simulink model "HvacCtrlrAirFlowDistbnFrnt"  (R2022b) (continued)
== system HvacCtrlrAirFlowDistbnFrnt == (continued)
  ClimaCfgForClimaCtrlMan1_ClimaCfgForClimaCtrlMan1/1 -> HvacCtrlrAirFlowDistbnFrnt_Runnable_sys/26(ClimaCfgForClimaCtrlMan1_ClimaCfgForClimaCtrlMan1_read)
  ClimaCfgForNrOfTSetg1_ClimaCfgForNrOfTSetg1/1 -> HvacCtrlrAirFlowDistbnFrnt_Runnable_sys/27(ClimaCfgForNrOfTSetg1_ClimaCfgForNrOfTSetg1_read)
  ClimaCtrlActvNgcc_ClimaCtrlActv/1 -> HvacCtrlrAirFlowDistbnFrnt_Runnable_sys/28(ClimaCtrlActvNgcc_ClimaCtrlActv_read)
  HmiCmptmtAirDistbnFrntForClima_HmiCmptmtAirDistbnFrntForClima/1 -> HvacCtrlrAirFlowDistbnFrnt_Runnable_sys/29(HmiCmptmtAirDistbnFrntForClima_HmiCmptmtAirDistbnFrntForClima_read)
  HmiCmptmtAirDistbnFrntForClima_HmiCmptmtAirDistbnFrntRiForClima/1 -> HvacCtrlrAirFlowDistbnFrnt_Runnable_sys/30(HmiCmptmtAirDistbnFrntForClima_HmiCmptmtAirDistbnFrntRiForClima_read)
  HmiCmptmtAirDistbnFrntForClima_HmiCmptmtAirDistbnReForClima/1 -> HvacCtrlrAirFlowDistbnFrnt_Runnable_sys/31(HmiCmptmtAirDistbnFrntForClima_HmiCmptmtAirDistbnReForClima_read)
  HmiCmptmtAirDistbnFrntForClima_HmiCmptmtAirDistbnReRiForClima/1 -> HvacCtrlrAirFlowDistbnFrnt_Runnable_sys/32(HmiCmptmtAirDistbnFrntForClima_HmiCmptmtAirDistbnReRiForClima_read)
  HvacAirMFlowEstimdForCtrlr_HvacAirMFlowEstimdForCtrlr/1 -> HvacCtrlrAirFlowDistbnFrnt_Runnable_sys/33(HvacAirMFlowEstimdForCtrlr_HvacAirMFlowEstimdForCtrlr_read)
  HvacCtrlrFanBoostReqLe_HvacCtrlrFanBoostReqLe/1 -> HvacCtrlrAirFlowDistbnFrnt_Runnable_sys/34(HvacCtrlrFanBoostReqLe_HvacCtrlrFanBoostReqLe_read)
  HvacCtrlrFanBoostReqRi_HvacCtrlrFanBoostReqRi/1 -> HvacCtrlrAirFlowDistbnFrnt_Runnable_sys/35(HvacCtrlrFanBoostReqRi_HvacCtrlrFanBoostReqRi_read)
  HvacCtrlrReqFrntForRowFirstForActrTLe_HvacCtrlrReqFrntForRowFirstForActrTLe/1 -> HvacCtrlrAirFlowDistbnFrnt_Runnable_sys/36(HvacCtrlrReqFrntForRowFirstForActrTLe_HvacCtrlrReqFrntForRowFirstForActrTLe_read)
  HvacCtrlrReqFrntForRowFirstForActrTRi_HvacCtrlrReqFrntForRowFirstForActrTRi/1 -> HvacCtrlrAirFlowDistbnFrnt_Runnable_sys/37(HvacCtrlrReqFrntForRowFirstForActrTRi_HvacCtrlrReqFrntForRowFirstForActrTRi_read)
  HvacCtrlrReqFrntForRowSecForActrTLe_HvacCtrlrReqFrntForRowSecForActrTLe/1 -> HvacCtrlrAirFlowDistbnFrnt_Runnable_sys/38(HvacCtrlrReqFrntForRowSecForActrTLe_HvacCtrlrReqFrntForRowSecForActrTLe_read)
  HvacCtrlrReqFrntForRowSecForActrTRi_HvacCtrlrReqFrntForRowSecForActrTRi/1 -> HvacCtrlrAirFlowDistbnFrnt_Runnable_sys/39(HvacCtrlrReqFrntForRowSecForActrTRi_HvacCtrlrReqFrntForRowSecForActrTRi_read)
  HvacFlowFrntActForDefrst_HvacFlowFrntActForDefrst/1 -> HvacCtrlrAirFlowDistbnFrnt_Runnable_sys/40(HvacFlowFrntActForDefrst_HvacFlowFrntActForDefrst_read)
  HvacFlowFrntActForRowFirstAtFlr_HvacFlowFrntActForRowFirstAtFlr/1 -> HvacCtrlrAirFlowDistbnFrnt_Runnable_sys/41(HvacFlowFrntActForRowFirstAtFlr_HvacFlowFrntActForRowFirstAtFlr_read)
  HvacFlowFrntActForRowFirstAtFlr_HvacFlowFrntActForRowFirstAtFlrLe/1 -> HvacCtrlrAirFlowDistbnFrnt_Runnable_sys/42(HvacFlowFrntActForRowFirstAtFlr_HvacFlowFrntActForRowFirstAtFlrLe_read)
  HvacFlowFrntActForRowFirstAtFlr_HvacFlowFrntActForRowFirstAtFlrRi/1 -> HvacCtrlrAirFlowDistbnFrnt_Runnable_sys/43(HvacFlowFrntActForRowFirstAtFlr_HvacFlowFrntActForRowFirstAtFlrRi_read)
  HvacFlowFrntActForRowFirstAtVent_HvacFlowFrntActForRowFirstAtVent/1 -> HvacCtrlrAirFlowDistbnFrnt_Runnable_sys/44(HvacFlowFrntActForRowFirstAtVent_HvacFlowFrntActForRowFirstAtVent_read)
  HvacFlowFrntActForRowFirstAtVent_HvacFlowFrntActForRowFirstAtVentLe/1 -> HvacCtrlrAirFlowDistbnFrnt_Runnable_sys/45(HvacFlowFrntActForRowFirstAtVent_HvacFlowFrntActForRowFirstAtVentLe_read)
  HvacFlowFrntActForRowFirstAtVent_HvacFlowFrntActForRowFirstAtVentRi/1 -> HvacCtrlrAirFlowDistbnFrnt_Runnable_sys/46(HvacFlowFrntActForRowFirstAtVent_HvacFlowFrntActForRowFirstAtVentRi_read)
  HvacFlowFrntActForRowFirstAtVent_HvacFlowFrntActForRowFirstAtVentSideLe/1 -> HvacCtrlrAirFlowDistbnFrnt_Runnable_sys/47(HvacFlowFrntActForRowFirstAtVent_HvacFlowFrntActForRowFirstAtVentSideLe_read)
  HvacFlowFrntActForRowFirstAtVent_HvacFlowFrntActForRowFirstAtVentSideRi/1 -> HvacCtrlrAirFlowDistbnFrnt_Runnable_sys/48(HvacFlowFrntActForRowFirstAtVent_HvacFlowFrntActForRowFirstAtVentSideRi_read)
  HvacFlowFrntActForRowSecAtFlr_HvacFlowFrntActForRowSecAtFlr/1 -> HvacCtrlrAirFlowDistbnFrnt_Runnable_sys/49(HvacFlowFrntActForRowSecAtFlr_HvacFlowFrntActForRowSecAtFlr_read)
  HvacFlowFrntActForRowSecAtFlr_HvacFlowFrntActForRowSecAtFlrLe/1 -> HvacCtrlrAirFlowDistbnFrnt_Runnable_sys/50(HvacFlowFrntActForRowSecAtFlr_HvacFlowFrntActForRowSecAtFlrLe_read)
  HvacFlowFrntActForRowSecAtFlr_HvacFlowFrntActForRowSecAtFlrRi/1 -> HvacCtrlrAirFlowDistbnFrnt_Runnable_sys/51(HvacFlowFrntActForRowSecAtFlr_HvacFlowFrntActForRowSecAtFlrRi_read)
  HvacFlowFrntActForRowSecAtVent_HvacFlowFrntActForRowSecAtVent/1 -> HvacCtrlrAirFlowDistbnFrnt_Runnable_sys/52(HvacFlowFrntActForRowSecAtVent_HvacFlowFrntActForRowSecAtVent_read)
  HvacFlowFrntActForRowSecAtVent_HvacFlowFrntActForRowSecAtVentLe/1 -> HvacCtrlrAirFlowDistbnFrnt_Runnable_sys/53(HvacFlowFrntActForRowSecAtVent_HvacFlowFrntActForRowSecAtVentLe_read)
  HvacFlowFrntActForRowSecAtVent_HvacFlowFrntActForRowSecAtVentRi/1 -> HvacCtrlrAirFlowDistbnFrnt_Runnable_sys/54(HvacFlowFrntActForRowSecAtVent_HvacFlowFrntActForRowSecAtVentRi_read)
  HvacFlowFrntReqForDefrst_HvacFlowFrntReqForDefrst/1 -> HvacCtrlrAirFlowDistbnFrnt_Runnable_sys/55(HvacFlowFrntReqForDefrst_HvacFlowFrntReqForDefrst_read)
  HvacFlowFrntReqForRowFirstAtFlr_HvacFlowFrntReqForRowFirstAtFlr/1 -> HvacCtrlrAirFlowDistbnFrnt_Runnable_sys/56(HvacFlowFrntReqForRowFirstAtFlr_HvacFlowFrntReqForRowFirstAtFlr_read)
  HvacFlowFrntReqForRowFirstAtFlr_HvacFlowFrntReqForRowFirstAtFlrLe/1 -> HvacCtrlrAirFlowDistbnFrnt_Runnable_sys/57(HvacFlowFrntReqForRowFirstAtFlr_HvacFlowFrntReqForRowFirstAtFlrLe_read)
  HvacFlowFrntReqForRowFirstAtFlr_HvacFlowFrntReqForRowFirstAtFlrRi/1 -> HvacCtrlrAirFlowDistbnFrnt_Runnable_sys/58(HvacFlowFrntReqForRowFirstAtFlr_HvacFlowFrntReqForRowFirstAtFlrRi_read)
  HvacFlowFrntReqForRowFirstAtVent_HvacFlowFrntReqForRowFirstAtVent/1 -> HvacCtrlrAirFlowDistbnFrnt_Runnable_sys/59(HvacFlowFrntReqForRowFirstAtVent_HvacFlowFrntReqForRowFirstAtVent_read)
  HvacFlowFrntReqForRowFirstAtVent_HvacFlowFrntReqForRowFirstAtVentLe/1 -> HvacCtrlrAirFlowDistbnFrnt_Runnable_sys/60(HvacFlowFrntReqForRowFirstAtVent_HvacFlowFrntReqForRowFirstAtVentLe_read)
  HvacFlowFrntReqForRowFirstAtVent_HvacFlowFrntReqForRowFirstAtVentLeMid/1 -> HvacCtrlrAirFlowDistbnFrnt_Runnable_sys/61(HvacFlowFrntReqForRowFirstAtVent_HvacFlowFrntReqForRowFirstAtVentLeMid_read)
  HvacFlowFrntReqForRowFirstAtVent_HvacFlowFrntReqForRowFirstAtVentLeSide/1 -> HvacCtrlrAirFlowDistbnFrnt_Runnable_sys/62(HvacFlowFrntReqForRowFirstAtVent_HvacFlowFrntReqForRowFirstAtVentLeSide_read)
  HvacFlowFrntReqForRowFirstAtVent_HvacFlowFrntReqForRowFirstAtVentRi/1 -> HvacCtrlrAirFlowDistbnFrnt_Runnable_sys/63(HvacFlowFrntReqForRowFirstAtVent_HvacFlowFrntReqForRowFirstAtVentRi_read)
  HvacFlowFrntReqForRowFirstAtVent_HvacFlowFrntReqForRowFirstAtVentRiMid/1 -> HvacCtrlrAirFlowDistbnFrnt_Runnable_sys/64(HvacFlowFrntReqForRowFirstAtVent_HvacFlowFrntReqForRowFirstAtVentRiMid_read)
  HvacFlowFrntReqForRowFirstAtVent_HvacFlowFrntReqForRowFirstAtVentRiSide/1 -> HvacCtrlrAirFlowDistbnFrnt_Runnable_sys/65(HvacFlowFrntReqForRowFirstAtVent_HvacFlowFrntReqForRowFirstAtVentRiSide_read)
  HvacFlowFrntReqForRowSecAtFlr_HvacFlowFrntReqForRowSecAtFlr/1 -> HvacCtrlrAirFlowDistbnFrnt_Runnable_sys/66(HvacFlowFrntReqForRowSecAtFlr_HvacFlowFrntReqForRowSecAtFlr_read)
  HvacFlowFrntReqForRowSecAtFlr_HvacFlowFrntReqForRowSecAtFlrLe/1 -> HvacCtrlrAirFlowDistbnFrnt_Runnable_sys/67(HvacFlowFrntReqForRowSecAtFlr_HvacFlowFrntReqForRowSecAtFlrLe_read)
  HvacFlowFrntReqForRowSecAtFlr_HvacFlowFrntReqForRowSecAtFlrRi/1 -> HvacCtrlrAirFlowDistbnFrnt_Runnable_sys/68(HvacFlowFrntReqForRowSecAtFlr_HvacFlowFrntReqForRowSecAtFlrRi_read)
  HvacFlowFrntReqForRowSecAtVent_HvacFlowFrntReqForRowSecAtVent/1 -> HvacCtrlrAirFlowDistbnFrnt_Runnable_sys/69(HvacFlowFrntReqForRowSecAtVent_HvacFlowFrntReqForRowSecAtVent_read)
  HvacFlowFrntReqForRowSecAtVent_HvacFlowFrntReqForRowSecAtVentLe/1 -> HvacCtrlrAirFlowDistbnFrnt_Runnable_sys/70(HvacFlowFrntReqForRowSecAtVent_HvacFlowFrntReqForRowSecAtVentLe_read)
  HvacFlowFrntReqForRowSecAtVent_HvacFlowFrntReqForRowSecAtVentRi/1 -> HvacCtrlrAirFlowDistbnFrnt_Runnable_sys/71(HvacFlowFrntReqForRowSecAtVent_HvacFlowFrntReqForRowSecAtVentRi_read)
  HvacFlrLeakReqForRowFirst_HvacFlrLeakReqForRowFirst/1 -> HvacCtrlrAirFlowDistbnFrnt_Runnable_sys/72(HvacFlrLeakReqForRowFirst_HvacFlrLeakReqForRowFirst_read)
  HvacFlrLeakReqForRowSec_HvacFlrLeakReqForRowSec/1 -> HvacCtrlrAirFlowDistbnFrnt_Runnable_sys/73(HvacFlrLeakReqForRowSec_HvacFlrLeakReqForRowSec_read)
  LdAdVal_CurrAdVal/1 -> HvacCtrlrAirFlowDistbnFrnt_Runnable_sys/74(LdAdVal_CurrAdVal_read)
  LdAdVal_ValidAdVal/1 -> HvacCtrlrAirFlowDistbnFrnt_Runnable_sys/75(LdAdVal_ValidAdVal_read)
  ManModDurgFanOffActv_ManModDurgFanOffActv/1 -> HvacCtrlrAirFlowDistbnFrnt_Runnable_sys/76(ManModDurgFanOffActv_ManModDurgFanOffActv_read)
  ManModeForRowFirstLeForModelDial_ManModeForRowFirstLeForModelDial/1 -> HvacCtrlrAirFlowDistbnFrnt_Runnable_sys/77(ManModeForRowFirstLeForModelDial_ManModeForRowFirstLeForModelDial_read)
  ManModeForRowFirstRiForModelDial_ManModeForRowFirstRiForModelDial/1 -> HvacCtrlrAirFlowDistbnFrnt_Runnable_sys/78(ManModeForRowFirstRiForModelDial_ManModeForRowFirstRiForModelDial_read)
  ManModeForRowSecLeForModelDial_ManModeForRowSecLeForModelDial/1 -> HvacCtrlrAirFlowDistbnFrnt_Runnable_sys/79(ManModeForRowSecLeForModelDial_ManModeForRowSecLeForModelDial_read)
  ManModeForRowSecRiForModelDial_ManModeForRowSecRiForModelDial/1 -> HvacCtrlrAirFlowDistbnFrnt_Runnable_sys/80(ManModeForRowSecRiForModelDial_ManModeForRowSecRiForModelDial_read)
  MaxReqReqdFrntForDefrst_MaxReqReqdFrntForDefrst/1 -> HvacCtrlrAirFlowDistbnFrnt_Runnable_sys/81(MaxReqReqdFrntForDefrst_MaxReqReqdFrntForDefrst_read)
  MaxReqReqdFrntForRowFirstAtFlr_MaxReqReqdFrntForRowFirstAtFlr/1 -> HvacCtrlrAirFlowDistbnFrnt_Runnable_sys/82(MaxReqReqdFrntForRowFirstAtFlr_MaxReqReqdFrntForRowFirstAtFlr_read)
  MaxReqReqdFrntForRowFirstAtFlr_MaxReqReqdFrntForRowFirstAtFlrLe/1 -> HvacCtrlrAirFlowDistbnFrnt_Runnable_sys/83(MaxReqReqdFrntForRowFirstAtFlr_MaxReqReqdFrntForRowFirstAtFlrLe_read)
  MaxReqReqdFrntForRowFirstAtFlr_MaxReqReqdFrntForRowFirstAtFlrRi/1 -> HvacCtrlrAirFlowDistbnFrnt_Runnable_sys/84(MaxReqReqdFrntForRowFirstAtFlr_MaxReqReqdFrntForRowFirstAtFlrRi_read)
  MaxReqReqdFrntForRowFirstAtVent_MaxReqReqdFrntForRowFirstAtVent/1 -> HvacCtrlrAirFlowDistbnFrnt_Runnable_sys/85(MaxReqReqdFrntForRowFirstAtVent_MaxReqReqdFrntForRowFirstAtVent_read)
  MaxReqReqdFrntForRowFirstAtVent_MaxReqReqdFrntForRowFirstAtVentLe/1 -> HvacCtrlrAirFlowDistbnFrnt_Runnable_sys/86(MaxReqReqdFrntForRowFirstAtVent_MaxReqReqdFrntForRowFirstAtVentLe_read)
  MaxReqReqdFrntForRowFirstAtVent_MaxReqReqdFrntForRowFirstAtVentRi/1 -> HvacCtrlrAirFlowDistbnFrnt_Runnable_sys/87(MaxReqReqdFrntForRowFirstAtVent_MaxReqReqdFrntForRowFirstAtVentRi_read)
  MaxReqReqdFrntForRowFirstAtVent_MaxReqReqdFrntForRowFirstAtVentSideLe/1 -> HvacCtrlrAirFlowDistbnFrnt_Runnable_sys/88(MaxReqReqdFrntForRowFirstAtVent_MaxReqReqdFrntForRowFirstAtVentSideLe_read)
  MaxReqReqdFrntForRowFirstAtVent_MaxReqReqdFrntForRowFirstAtVentSideRi/1 -> HvacCtrlrAirFlowDistbnFrnt_Runnable_sys/89(MaxReqReqdFrntForRowFirstAtVent_MaxReqReqdFrntForRowFirstAtVentSideRi_read)
  MaxReqReqdFrntForRowSecAtFlr_MaxReqReqdFrntForRowSecAtFlr/1 -> HvacCtrlrAirFlowDistbnFrnt_Runnable_sys/90(MaxReqReqdFrntForRowSecAtFlr_MaxReqReqdFrntForRowSecAtFlr_read)
  MaxReqReqdFrntForRowSecAtFlr_MaxReqReqdFrntForRowSecAtFlrLe/1 -> HvacCtrlrAirFlowDistbnFrnt_Runnable_sys/91(MaxReqReqdFrntForRowSecAtFlr_MaxReqReqdFrntForRowSecAtFlrLe_read)
  MaxReqReqdFrntForRowSecAtFlr_MaxReqReqdFrntForRowSecAtFlrRi/1 -> HvacCtrlrAirFlowDistbnFrnt_Runnable_sys/92(MaxReqReqdFrntForRowSecAtFlr_MaxReqReqdFrntForRowSecAtFlrRi_read)
  MaxReqReqdFrntForRowSecAtVent_MaxReqReqdFrntForRowSecAtVent/1 -> HvacCtrlrAirFlowDistbnFrnt_Runnable_sys/93(MaxReqReqdFrntForRowSecAtVent_MaxReqReqdFrntForRowSecAtVent_read)
  MaxReqReqdFrntForRowSecAtVent_MaxReqReqdFrntForRowSecAtVentLe/1 -> HvacCtrlrAirFlowDistbnFrnt_Runnable_sys/94(MaxReqReqdFrntForRowSecAtVent_MaxReqReqdFrntForRowSecAtVentLe_read)
  MaxReqReqdFrntForRowSecAtVent_MaxReqReqdFrntForRowSecAtVentRi/1 -> HvacCtrlrAirFlowDistbnFrnt_Runnable_sys/95(MaxReqReqdFrntForRowSecAtVent_MaxReqReqdFrntForRowSecAtVentRi_read)
  NotAllwdForMaxReqReqdForRowSecAtVent_NotAllwdForMaxReqReqdForRowSecAtVent/1 -> HvacCtrlrAirFlowDistbnFrnt_Runnable_sys/96(NotAllwdForMaxReqReqdForRowSecAtVent_NotAllwdForMaxReqReqdForRowSecAtVent_read)
  VehSpdLgtForClima_VehSpdLgtForClima/1 -> HvacCtrlrAirFlowDistbnFrnt_Runnable_sys/97(VehSpdLgtForClima_VehSpdLgtForClima_read)
  VehSunLoadAtRowFirstLeFild_VehSunLoadAtRowFirstLeFild/1 -> HvacCtrlrAirFlowDistbnFrnt_Runnable_sys/98(VehSunLoadAtRowFirstLeFild_VehSunLoadAtRowFirstLeFild_read)
  VehSunLoadAtRowFirstRiFild_VehSunLoadAtRowFirstRiFild/1 -> HvacCtrlrAirFlowDistbnFrnt_Runnable_sys/99(VehSunLoadAtRowFirstRiFild_VehSunLoadAtRowFirstRiFild_read)
  ActrFrntReqForRowFirstLeModDistbn_ActrFrntReqForRowFirstLeModDistbn/1 -> HvacCtrlrAirFlowDistbnFrnt_Runnable_sys/100(ActrFrntReqForRowFirstLeModDistbn_ActrFrntReqForRowFirstLeModDistbn_read)
  ActrFrntReqForRowFirstLeModDistbn_ActrFrntReqForRowFirstLeModDistbn_IsUpdated/1 -> HvacCtrlrAirFlowDistbnFrnt_Runnable_sys/101(ActrFrntReqForRowFirstLeModDistbn_ActrFrntReqForRowFirstLeModDistbn_IsUpdated_read)
  ActrFrntReqForRowFirstModDistbn_ActrFrntReqForRowFirstModDistbn/1 -> HvacCtrlrAirFlowDistbnFrnt_Runnable_sys/102(ActrFrntReqForRowFirstModDistbn_ActrFrntReqForRowFirstModDistbn_read)
  ActrFrntReqForRowFirstModDistbn_ActrFrntReqForRowFirstModDistbn_IsUpdated/1 -> HvacCtrlrAirFlowDistbnFrnt_Runnable_sys/103(ActrFrntReqForRowFirstModDistbn_ActrFrntReqForRowFirstModDistbn_IsUpdated_read)
  ActrFrntReqForRowSecModDistbnLe_ActrFrntReqForRowSecModDistbnLe/1 -> HvacCtrlrAirFlowDistbnFrnt_Runnable_sys/104(ActrFrntReqForRowSecModDistbnLe_ActrFrntReqForRowSecModDistbnLe_read)
  ActrFrntReqForRowSecModDistbnLe_ActrFrntReqForRowSecModDistbnLe_IsUpdated/1 -> HvacCtrlrAirFlowDistbnFrnt_Runnable_sys/105(ActrFrntReqForRowSecModDistbnLe_ActrFrntReqForRowSecModDistbnLe_IsUpdated_read)
  ActrFrntReqForRowSecModDistbnRi_ActrFrntReqForRowSecModDistbnRi/1 -> HvacCtrlrAirFlowDistbnFrnt_Runnable_sys/106(ActrFrntReqForRowSecModDistbnRi_ActrFrntReqForRowSecModDistbnRi_read)
  ActrFrntReqForRowSecModDistbnRi_ActrFrntReqForRowSecModDistbnRi_IsUpdated/1 -> HvacCtrlrAirFlowDistbnFrnt_Runnable_sys/107(ActrFrntReqForRowSecModDistbnRi_ActrFrntReqForRowSecModDistbnRi_IsUpdated_read)
  ActrPosnFrntActForRowFirstModDistbnLe_ActrPosnFrntActForRowFirstModDistbnLe/1 -> HvacCtrlrAirFlowDistbnFrnt_Runnable_sys/108(ActrPosnFrntActForRowFirstModDistbnLe_ActrPosnFrntActForRowFirstModDistbnLe_read)
  ActrReqForModDistbnRe_ActrReqForModDistbnRe/1 -> HvacCtrlrAirFlowDistbnFrnt_Runnable_sys/109(ActrReqForModDistbnRe_ActrReqForModDistbnRe_read)
  ActrReqForModDistbnRe_ActrReqForModDistbnRe_IsUpdated/1 -> HvacCtrlrAirFlowDistbnFrnt_Runnable_sys/110(ActrReqForModDistbnRe_ActrReqForModDistbnRe_IsUpdated_read)
  ClimaAtRowSecActvNgcc_ClimaAtRowSecActv/1 -> HvacCtrlrAirFlowDistbnFrnt_Runnable_sys/111(ClimaAtRowSecActvNgcc_ClimaAtRowSecActv_read)
  ClimaAtRowSecActvNgcc_ClimaAtRowSecActvLe/1 -> HvacCtrlrAirFlowDistbnFrnt_Runnable_sys/112(ClimaAtRowSecActvNgcc_ClimaAtRowSecActvLe_read)
  ClimaAtRowSecActvNgcc_ClimaAtRowSecActvRi/1 -> HvacCtrlrAirFlowDistbnFrnt_Runnable_sys/113(ClimaAtRowSecActvNgcc_ClimaAtRowSecActvRi_read)
  ClimaCfgForHvacRePrsnt1_ClimaCfgForHvacRePrsnt1/1 -> HvacCtrlrAirFlowDistbnFrnt_Runnable_sys/114(ClimaCfgForHvacRePrsnt1_ClimaCfgForHvacRePrsnt1_read)
  ClimaCfgReqVersionReplace_ClimaCfgReqVersionReplace/1 -> HvacCtrlrAirFlowDistbnFrnt_Runnable_sys/115(ClimaCfgReqVersionReplace_ClimaCfgReqVersionReplace_read)
  ManModeForRowThrdForModelDial_ManModeForRowThrdForModelDial/1 -> HvacCtrlrAirFlowDistbnFrnt_Runnable_sys/116(ManModeForRowThrdForModelDial_ManModeForRowThrdForModelDial_read)
  PrkgClimaOnReq_PrkgClimaOnReq/1 -> HvacCtrlrAirFlowDistbnFrnt_Runnable_sys/117(PrkgClimaOnReq_PrkgClimaOnReq_read)
  HvacCtrlrAirFlowDistbnFrnt_Runnable_sys/1(HvacAirMFlowReqMaxAvl_HvacAirMFlowReqMaxAvl_write) -> HvacAirMFlowReqMaxAvl_HvacAirMFlowReqMaxAvl/1
  HvacCtrlrAirFlowDistbnFrnt_Runnable_sys/2(HvacCtrlrReqFrntForRowFirstForActrDefrst_HvacCtrlrReqFrntForRowFirstForActrDefrst_write) -> HvacCtrlrReqFrntForRowFirstForActrDefrst_HvacCtrlrReqFrntForRowFirstForActrDefrst/1
  HvacCtrlrAirFlowDistbnFrnt_Runnable_sys/3(HvacCtrlrReqFrntForRowFirstForActrFlr_HvacCtrlrReqFrntForRowFirstForActrFlr_write) -> HvacCtrlrReqFrntForRowFirstForActrFlr_HvacCtrlrReqFrntForRowFirstForActrFlr/1
  HvacCtrlrAirFlowDistbnFrnt_Runnable_sys/4(HvacCtrlrReqFrntForRowFirstForActrFlr_HvacCtrlrReqFrntForRowFirstForActrFlrLe_write) -> HvacCtrlrReqFrntForRowFirstForActrFlr_HvacCtrlrReqFrntForRowFirstForActrFlrLe/1
  HvacCtrlrAirFlowDistbnFrnt_Runnable_sys/5(HvacCtrlrReqFrntForRowFirstForActrFlr_HvacCtrlrReqFrntForRowFirstForActrFlrRi_write) -> HvacCtrlrReqFrntForRowFirstForActrFlr_HvacCtrlrReqFrntForRowFirstForActrFlrRi/1
  HvacCtrlrAirFlowDistbnFrnt_Runnable_sys/6(HvacCtrlrReqFrntForRowFirstForActrMod_HvacCtrlrReqFrntForRowFirstForActrMod_write) -> HvacCtrlrReqFrntForRowFirstForActrMod_HvacCtrlrReqFrntForRowFirstForActrMod/1
  HvacCtrlrAirFlowDistbnFrnt_Runnable_sys/7(HvacCtrlrReqFrntForRowFirstForActrVent_HvacCtrlrReqFrntForRowFirstForActrVent_write) -> HvacCtrlrReqFrntForRowFirstForActrVent_HvacCtrlrReqFrntForRowFirstForActrVent/1
  HvacCtrlrAirFlowDistbnFrnt_Runnable_sys/8(HvacCtrlrReqFrntForRowFirstForActrVent_HvacCtrlrReqFrntForRowFirstForActrVentLe_write) -> HvacCtrlrReqFrntForRowFirstForActrVent_HvacCtrlrReqFrntForRowFirstForActrVentLe/1
  HvacCtrlrAirFlowDistbnFrnt_Runnable_sys/9(HvacCtrlrReqFrntForRowFirstForActrVent_HvacCtrlrReqFrntForRowFirstForActrVentRi_write) -> HvacCtrlrReqFrntForRowFirstForActrVent_HvacCtrlrReqFrntForRowFirstForActrVentRi/1
  HvacCtrlrAirFlowDistbnFrnt_Runnable_sys/10(HvacCtrlrReqFrntForRowFirstForActrVent_HvacCtrlrReqFrntForRowFirstForActrVentSideLe_write) -> HvacCtrlrReqFrntForRowFirstForActrVent_HvacCtrlrReqFrntForRowFirstForActrVentSideLe/1
  HvacCtrlrAirFlowDistbnFrnt_Runnable_sys/11(HvacCtrlrReqFrntForRowFirstForActrVent_HvacCtrlrReqFrntForRowFirstForActrVentSideRi_write) -> HvacCtrlrReqFrntForRowFirstForActrVent_HvacCtrlrReqFrntForRowFirstForActrVentSideRi/1
  HvacCtrlrAirFlowDistbnFrnt_Runnable_sys/12(HvacCtrlrReqFrntForRowFirstLeForActrMod_HvacCtrlrReqFrntForRowFirstLeForActrMod_write) -> HvacCtrlrReqFrntForRowFirstLeForActrMod_HvacCtrlrReqFrntForRowFirstLeForActrMod/1
  HvacCtrlrAirFlowDistbnFrnt_Runnable_sys/13(HvacCtrlrReqFrntForRowSecForActrFlr_HvacCtrlrReqFrntForRowSecForActrFlr_write) -> HvacCtrlrReqFrntForRowSecForActrFlr_HvacCtrlrReqFrntForRowSecForActrFlr/1
  HvacCtrlrAirFlowDistbnFrnt_Runnable_sys/14(HvacCtrlrReqFrntForRowSecForActrFlr_HvacCtrlrReqFrntForRowSecForActrFlrLe_write) -> HvacCtrlrReqFrntForRowSecForActrFlr_HvacCtrlrReqFrntForRowSecForActrFlrLe/1
  HvacCtrlrAirFlowDistbnFrnt_Runnable_sys/15(HvacCtrlrReqFrntForRowSecForActrFlr_HvacCtrlrReqFrntForRowSecForActrFlrRi_write) -> HvacCtrlrReqFrntForRowSecForActrFlr_HvacCtrlrReqFrntForRowSecForActrFlrRi/1
  HvacCtrlrAirFlowDistbnFrnt_Runnable_sys/16(HvacCtrlrReqFrntForRowSecForActrModLe_HvacCtrlrReqFrntForRowSecForActrModLe_write) -> HvacCtrlrReqFrntForRowSecForActrModLe_HvacCtrlrReqFrntForRowSecForActrModLe/1
  HvacCtrlrAirFlowDistbnFrnt_Runnable_sys/17(HvacCtrlrReqFrntForRowSecForActrModRi_HvacCtrlrReqFrntForRowSecForActrModRi_write) -> HvacCtrlrReqFrntForRowSecForActrModRi_HvacCtrlrReqFrntForRowSecForActrModRi/1
  HvacCtrlrAirFlowDistbnFrnt_Runnable_sys/18(HvacCtrlrReqFrntForRowSecForActrVent_HvacCtrlrReqFrntForRowSecForActrVent_write) -> HvacCtrlrReqFrntForRowSecForActrVent_HvacCtrlrReqFrntForRowSecForActrVent/1
  HvacCtrlrAirFlowDistbnFrnt_Runnable_sys/19(OvrdForMaxReqForActrForDefrst_OvrdForMaxReqForActrForDefrst_write) -> OvrdForMaxReqForActrForDefrst_OvrdForMaxReqForActrForDefrst/1
  HvacCtrlrAirFlowDistbnFrnt_Runnable_sys/20(OvrdForMaxReqForActrForRowFirstAtFlr_OvrdForMaxReqForActrForRowFirstAtFlr_write) -> OvrdForMaxReqForActrForRowFirstAtFlr_OvrdForMaxReqForActrForRowFirstAtFlr/1
  OvrdForMaxReqForActrForRowFirstAtFlr_OvrdForMaxReqForActrForRowFirstAtFlrLe_merge/1 -> OvrdForMaxReqForActrForRowFirstAtFlr_OvrdForMaxReqForActrForRowFirstAtFlrLe/1
  OvrdForMaxReqForActrForRowFirstAtFlr_OvrdForMaxReqForActrForRowFirstAtFlrRi_merge/1 -> OvrdForMaxReqForActrForRowFirstAtFlr_OvrdForMaxReqForActrForRowFirstAtFlrRi/1
  HvacCtrlrAirFlowDistbnFrnt_Runnable_sys/23(OvrdForMaxReqForActrForRowFirstAtVent_OvrdForMaxReqForActrForRowFirstAtVent_write) -> OvrdForMaxReqForActrForRowFirstAtVent_OvrdForMaxReqForActrForRowFirstAtVent/1
  OvrdForMaxReqForActrForRowFirstAtVent_OvrdForMaxReqForActrForRowFirstAtVentLe_merge/1 -> OvrdForMaxReqForActrForRowFirstAtVent_OvrdForMaxReqForActrForRowFirstAtVentLe/1
  OvrdForMaxReqForActrForRowFirstAtVent_OvrdForMaxReqForActrForRowFirstAtVentRi_merge/1 -> OvrdForMaxReqForActrForRowFirstAtVent_OvrdForMaxReqForActrForRowFirstAtVentRi/1
  OvrdForMaxReqForActrForRowFirstAtVent_OvrdForMaxReqForActrForRowFirstAtVentSideLe_merge/1 -> OvrdForMaxReqForActrForRowFirstAtVent_OvrdForMaxReqForActrForRowFirstAtVentSideLe/1
  OvrdForMaxReqForActrForRowFirstAtVent_OvrdForMaxReqForActrForRowFirstAtVentSideRi_merge/1 -> OvrdForMaxReqForActrForRowFirstAtVent_OvrdForMaxReqForActrForRowFirstAtVentSideRi/1
  HvacCtrlrAirFlowDistbnFrnt_Runnable_sys/28(OvrdForMaxReqForActrForRowSecAtFlr_OvrdForMaxReqForActrForRowSecAtFlr_write) -> OvrdForMaxReqForActrForRowSecAtFlr_OvrdForMaxReqForActrForRowSecAtFlr/1
  OvrdForMaxReqForActrForRowSecAtFlr_OvrdForMaxReqForActrForRowSecAtFlrLe_merge/1 -> OvrdForMaxReqForActrForRowSecAtFlr_OvrdForMaxReqForActrForRowSecAtFlrLe/1
  OvrdForMaxReqForActrForRowSecAtFlr_OvrdForMaxReqForActrForRowSecAtFlrRi_merge/1 -> OvrdForMaxReqForActrForRowSecAtFlr_OvrdForMaxReqForActrForRowSecAtFlrRi/1
  HvacCtrlrAirFlowDistbnFrnt_Runnable_sys/31(OvrdForMaxReqForActrForRowSecAtVent_OvrdForMaxReqForActrForRowSecAtVent_write) -> OvrdForMaxReqForActrForRowSecAtVent_OvrdForMaxReqForActrForRowSecAtVent/1
  OvrdForMaxReqForActrForRowSecAtVent_OvrdForMaxReqForActrForRowSecAtVentLe_merge/1 -> OvrdForMaxReqForActrForRowSecAtVent_OvrdForMaxReqForActrForRowSecAtVentLe/1
  OvrdForMaxReqForActrForRowSecAtVent_OvrdForMaxReqForActrForRowSecAtVentRi_merge/1 -> OvrdForMaxReqForActrForRowSecAtVent_OvrdForMaxReqForActrForRowSecAtVentRi/1
  HvacCtrlrAirFlowDistbnFrnt_Runnable_sys/34(ResrvdSigForECM4_ResrvdSigForECM4_write) -> ResrvdSigForECM4_ResrvdSigForECM4/1
  HvacCtrlrAirFlowDistbnFrnt_Runnable_sys/33(OvrdForMaxReqForActrForRowSecAtVent_OvrdForMaxReqForActrForRowSecAtVentRi_write) -> [OvrdForMaxReqForActrForRowSecAtVent_OvrdForMax_60ad04dd0383d372]
  [OvrdForMaxReqForActrForRowSecAtVent_OvrdForMax_60ad04dd0383d372] -> OvrdForMaxReqForActrForRowSecAtVent_OvrdForMaxReqForActrForRowSecAtVentRi_merge/2
  HvacCtrlrAirFlowDistbnFrnt_Init/10(OvrdForMaxReqForActrForRowSecAtVent_OvrdForMaxReqForActrForRowSecAtVentRi_write) -> [OvrdForMaxReqForActrForRowSecAtVent_OvrdForMax_dc57e3a46c933c58]
  [OvrdForMaxReqForActrForRowSecAtVent_OvrdForMax_dc57e3a46c933c58] -> OvrdForMaxReqForActrForRowSecAtVent_OvrdForMaxReqForActrForRowSecAtVentRi_merge/1
  HvacCtrlrAirFlowDistbnFrnt_Runnable_sys/32(OvrdForMaxReqForActrForRowSecAtVent_OvrdForMaxReqForActrForRowSecAtVentLe_write) -> [OvrdForMaxReqForActrForRowSecAtVent_OvrdForMax_2f1dbdbaa78c8bf5]
  [OvrdForMaxReqForActrForRowSecAtVent_OvrdForMax_2f1dbdbaa78c8bf5] -> OvrdForMaxReqForActrForRowSecAtVent_OvrdForMaxReqForActrForRowSecAtVentLe_merge/2
  HvacCtrlrAirFlowDistbnFrnt_Init/9(OvrdForMaxReqForActrForRowSecAtVent_OvrdForMaxReqForActrForRowSecAtVentLe_write) -> [OvrdForMaxReqForActrForRowSecAtVent_OvrdForMax_3cb761358e7e1665]
  [OvrdForMaxReqForActrForRowSecAtVent_OvrdForMax_3cb761358e7e1665] -> OvrdForMaxReqForActrForRowSecAtVent_OvrdForMaxReqForActrForRowSecAtVentLe_merge/1
  HvacCtrlrAirFlowDistbnFrnt_Runnable_sys/30(OvrdForMaxReqForActrForRowSecAtFlr_OvrdForMaxReqForActrForRowSecAtFlrRi_write) -> [OvrdForMaxReqForActrForRowSecAtFlr_OvrdForMaxR_5db6c18a7a1e3353]
  [OvrdForMaxReqForActrForRowSecAtFlr_OvrdForMaxR_5db6c18a7a1e3353] -> OvrdForMaxReqForActrForRowSecAtFlr_OvrdForMaxReqForActrForRowSecAtFlrRi_merge/2
  HvacCtrlrAirFlowDistbnFrnt_Init/8(OvrdForMaxReqForActrForRowSecAtFlr_OvrdForMaxReqForActrForRowSecAtFlrRi_write) -> [OvrdForMaxReqForActrForRowSecAtFlr_OvrdForMaxR_e391f3db894718d1]
  [OvrdForMaxReqForActrForRowSecAtFlr_OvrdForMaxR_e391f3db894718d1] -> OvrdForMaxReqForActrForRowSecAtFlr_OvrdForMaxReqForActrForRowSecAtFlrRi_merge/1
  HvacCtrlrAirFlowDistbnFrnt_Runnable_sys/29(OvrdForMaxReqForActrForRowSecAtFlr_OvrdForMaxReqForActrForRowSecAtFlrLe_write) -> [OvrdForMaxReqForActrForRowSecAtFlr_OvrdForMaxR_bc7d79b631d9d563]
  [OvrdForMaxReqForActrForRowSecAtFlr_OvrdForMaxR_bc7d79b631d9d563] -> OvrdForMaxReqForActrForRowSecAtFlr_OvrdForMaxReqForActrForRowSecAtFlrLe_merge/2
  HvacCtrlrAirFlowDistbnFrnt_Init/7(OvrdForMaxReqForActrForRowSecAtFlr_OvrdForMaxReqForActrForRowSecAtFlrLe_write) -> [OvrdForMaxReqForActrForRowSecAtFlr_OvrdForMaxR_e6c435a498c64cbf]
  [OvrdForMaxReqForActrForRowSecAtFlr_OvrdForMaxR_e6c435a498c64cbf] -> OvrdForMaxReqForActrForRowSecAtFlr_OvrdForMaxReqForActrForRowSecAtFlrLe_merge/1
  HvacCtrlrAirFlowDistbnFrnt_Runnable_sys/27(OvrdForMaxReqForActrForRowFirstAtVent_OvrdForMaxReqForActrForRowFirstAtVentSideRi_write) -> [OvrdForMaxReqForActrForRowFirstAtVent_OvrdForM_5af40f56349c4786]
  [OvrdForMaxReqForActrForRowFirstAtVent_OvrdForM_5af40f56349c4786] -> OvrdForMaxReqForActrForRowFirstAtVent_OvrdForMaxReqForActrForRowFirstAtVentSideRi_merge/2
  HvacCtrlrAirFlowDistbnFrnt_Init/6(OvrdForMaxReqForActrForRowFirstAtVent_OvrdForMaxReqForActrForRowFirstAtVentSideRi_write) -> [OvrdForMaxReqForActrForRowFirstAtVent_OvrdForM_7dc9fe321c35ff24]
  [OvrdForMaxReqForActrForRowFirstAtVent_OvrdForM_7dc9fe321c35ff24] -> OvrdForMaxReqForActrForRowFirstAtVent_OvrdForMaxReqForActrForRowFirstAtVentSideRi_merge/1
  HvacCtrlrAirFlowDistbnFrnt_Runnable_sys/26(OvrdForMaxReqForActrForRowFirstAtVent_OvrdForMaxReqForActrForRowFirstAtVentSideLe_write) -> [OvrdForMaxReqForActrForRowFirstAtVent_OvrdForM_798a63b174726617]
  [OvrdForMaxReqForActrForRowFirstAtVent_OvrdForM_798a63b174726617] -> OvrdForMaxReqForActrForRowFirstAtVent_OvrdForMaxReqForActrForRowFirstAtVentSideLe_merge/2
  HvacCtrlrAirFlowDistbnFrnt_Init/5(OvrdForMaxReqForActrForRowFirstAtVent_OvrdForMaxReqForActrForRowFirstAtVentSideLe_write) -> [OvrdForMaxReqForActrForRowFirstAtVent_OvrdForM_2c9be2f0f81f1eeb]
  [OvrdForMaxReqForActrForRowFirstAtVent_OvrdForM_2c9be2f0f81f1eeb] -> OvrdForMaxReqForActrForRowFirstAtVent_OvrdForMaxReqForActrForRowFirstAtVentSideLe_merge/1
  HvacCtrlrAirFlowDistbnFrnt_Runnable_sys/25(OvrdForMaxReqForActrForRowFirstAtVent_OvrdForMaxReqForActrForRowFirstAtVentRi_write) -> [OvrdForMaxReqForActrForRowFirstAtVent_OvrdForM_21573a63da932dcc]
  [OvrdForMaxReqForActrForRowFirstAtVent_OvrdForM_21573a63da932dcc] -> OvrdForMaxReqForActrForRowFirstAtVent_OvrdForMaxReqForActrForRowFirstAtVentRi_merge/2
  HvacCtrlrAirFlowDistbnFrnt_Init/4(OvrdForMaxReqForActrForRowFirstAtVent_OvrdForMaxReqForActrForRowFirstAtVentRi_write) -> [OvrdForMaxReqForActrForRowFirstAtVent_OvrdForM_40f71520e326bfc9]
  [OvrdForMaxReqForActrForRowFirstAtVent_OvrdForM_40f71520e326bfc9] -> OvrdForMaxReqForActrForRowFirstAtVent_OvrdForMaxReqForActrForRowFirstAtVentRi_merge/1
  HvacCtrlrAirFlowDistbnFrnt_Runnable_sys/24(OvrdForMaxReqForActrForRowFirstAtVent_OvrdForMaxReqForActrForRowFirstAtVentLe_write) -> [OvrdForMaxReqForActrForRowFirstAtVent_OvrdForM_0ebbdfc67204fadc]
  [OvrdForMaxReqForActrForRowFirstAtVent_OvrdForM_0ebbdfc67204fadc] -> OvrdForMaxReqForActrForRowFirstAtVent_OvrdForMaxReqForActrForRowFirstAtVentLe_merge/2
  HvacCtrlrAirFlowDistbnFrnt_Init/3(OvrdForMaxReqForActrForRowFirstAtVent_OvrdForMaxReqForActrForRowFirstAtVentLe_write) -> [OvrdForMaxReqForActrForRowFirstAtVent_OvrdForM_90d38fab5beaf7f2]
  [OvrdForMaxReqForActrForRowFirstAtVent_OvrdForM_90d38fab5beaf7f2] -> OvrdForMaxReqForActrForRowFirstAtVent_OvrdForMaxReqForActrForRowFirstAtVentLe_merge/1
  HvacCtrlrAirFlowDistbnFrnt_Runnable_sys/22(OvrdForMaxReqForActrForRowFirstAtFlr_OvrdForMaxReqForActrForRowFirstAtFlrRi_write) -> [OvrdForMaxReqForActrForRowFirstAtFlr_OvrdForMa_93df92be7538b4c6]
  [OvrdForMaxReqForActrForRowFirstAtFlr_OvrdForMa_93df92be7538b4c6] -> OvrdForMaxReqForActrForRowFirstAtFlr_OvrdForMaxReqForActrForRowFirstAtFlrRi_merge/2
  HvacCtrlrAirFlowDistbnFrnt_Init/2(OvrdForMaxReqForActrForRowFirstAtFlr_OvrdForMaxReqForActrForRowFirstAtFlrRi_write) -> [OvrdForMaxReqForActrForRowFirstAtFlr_OvrdForMa_2897c0a2ffbaf3b1]
  [OvrdForMaxReqForActrForRowFirstAtFlr_OvrdForMa_2897c0a2ffbaf3b1] -> OvrdForMaxReqForActrForRowFirstAtFlr_OvrdForMaxReqForActrForRowFirstAtFlrRi_merge/1
  HvacCtrlrAirFlowDistbnFrnt_Runnable_sys/21(OvrdForMaxReqForActrForRowFirstAtFlr_OvrdForMaxReqForActrForRowFirstAtFlrLe_write) -> [OvrdForMaxReqForActrForRowFirstAtFlr_OvrdForMa_1d1e6167545e3c8f]
  [OvrdForMaxReqForActrForRowFirstAtFlr_OvrdForMa_1d1e6167545e3c8f] -> OvrdForMaxReqForActrForRowFirstAtFlr_OvrdForMaxReqForActrForRowFirstAtFlrLe_merge/2
  HvacCtrlrAirFlowDistbnFrnt_Init/1(OvrdForMaxReqForActrForRowFirstAtFlr_OvrdForMaxReqForActrForRowFirstAtFlrLe_write) -> [OvrdForMaxReqForActrForRowFirstAtFlr_OvrdForMa_2ba4c4c650998548]
  [OvrdForMaxReqForActrForRowFirstAtFlr_OvrdForMa_2ba4c4c650998548] -> OvrdForMaxReqForActrForRowFirstAtFlr_OvrdForMaxReqForActrForRowFirstAtFlrLe_merge/1
  HvacCtrlrAirFlowDistbnFrnt_Runnable/1 -> HvacCtrlrAirFlowDistbnFrnt_Runnable_sys/Trigger
